simulink model "wlan_phy_rx_pmd"  (Simulink 7.8)
settings: Solver=VariableStepDiscrete  SolverMode=SingleTasking  StartTime=0.0  StopTime=simtime  RelTol=1e-3
PostLoadFcn:
  | wlan_phy_rx_init
InitFcn:
  | wlan_phy_rx_init
StartFcn:
  | wlan_phy_rx_init
values from neighbouring files (symbols this model uses):
  barker_seq20 = 0.95 * circshift(barker_seq20, [0 4]) ./ max(abs(barker_seq20))    [wlan_phy_rx_init.m]
  bit_scrambler_lfsr_bytes = bi2de(reshape(repmat(scr, 1, 8), 8, 127)', 'left-msb')    [wlan_phy_rx_init.m]
  deinterleave_ROM = [deinterleave_ROM interleave_64QAM zeros(1, 512-length(interleave_64QAM))]    [wlan_phy_rx_init.m]
  longCorr_coef_i = [3*real(LTS_corr)]    [wlan_phy_rx_init.m]
  longCorr_coef_q = [3*imag(LTS_corr)]    [wlan_phy_rx_init.m]
  MAX_CP_LEN = 32    [wlan_phy_rx_init.m]
  MAX_NCBPS = 288    [wlan_phy_rx_init.m]
  MAX_NUM_BYTES = 4096    [wlan_phy_rx_init.m]
  MAX_NUM_SC = 64    [wlan_phy_rx_init.m]
  MAX_NUM_SYMS = 600    [wlan_phy_rx_init.m]
  n_bits_preFFT_sampBuff = ceil(log2(4*MAX_NUM_SC))    [wlan_phy_rx_init.m]
  NCBPS_16QAM = 192    [wlan_phy_rx_init.m]
  NCBPS_64QAM = 288    [wlan_phy_rx_init.m]
  NCBPS_BPSK = 48    [wlan_phy_rx_init.m]
  NCBPS_QPSK = 96    [wlan_phy_rx_init.m]
  NDBPS_16QAM12 = 96    [wlan_phy_rx_init.m]
  NDBPS_16QAM34 = 144    [wlan_phy_rx_init.m]
  NDBPS_64QAM23 = 192    [wlan_phy_rx_init.m]
  NDBPS_64QAM34 = 216    [wlan_phy_rx_init.m]
  NDBPS_BPSK12 = 24    [wlan_phy_rx_init.m]
  NDBPS_BPSK34 = 36    [wlan_phy_rx_init.m]
  NDBPS_QPSK12 = 48    [wlan_phy_rx_init.m]
  NDBPS_QPSK34 = 72    [wlan_phy_rx_init.m]
  paylod_vec_samp_time = 8    [wlan_phy_rx_init.m]
  PHY_MIN_PKT_LEN = 14    [wlan_phy_rx_init.m]
  scr_ind_rev = zeros(1,128)    [wlan_phy_rx_init.m]
undefined symbols (not defined in the file or in its folder): gcbh, xlBlockLoadCallback, xlGetNormalizedPeriod, xlipgetpartsetting
libraries: xbsIndex_r4 x3519, simulink x1, xbsEDKLib_r4 x1
(part 8 of 21)

== system wlan_phy_rx_pmd/Chan Est & EQ/Chan Est/Q Estimate Buffer ==
  note: data
  Est_Data : Inport
  Est_Addr : Inport  Port=2
  Est_WE : Inport  Port=3
  First Sym : Inport  Port=4
  AddSub2 : xbsIndex_r4/AddSub  mode=Addition  use_carryin=off  use_carryout=off  en=off  latency=0  precision=User Defined  arith_type="Signed  (2's comp)"  n_bits=18  bin_pt=15  quantization=Truncate  overflow=Flag as error  dbl_ovrd=off  use_behavioral_HDL=on  hw_selection=Fabric  pipelined=off  use_rpm=on  block_type=addsub
      settings: xl_area=[0, 0, 0, 0, 0, 0, 0]  block_version=VER_STRING_GOES_HERE
  Constant Read State : xbsIndex_r4/Constant  const=0  gui_display_data_type=Fixed-point  arith_type=Boolean  n_bits=1  bin_pt=0  preci_type=Single  exp_width=8  frac_width=24  explicit_period=off  period=1  equ=P=C  opselect=C  inp2=PCIN>>17  opr=+  inp1=P  carry=CIN  dbl_ovrd=off  block_type=constant
      settings: block_version=VER_STRING_GOES_HERE
  Constant4 : xbsIndex_r4/Constant  const=0  gui_display_data_type=Fixed-point  arith_type=Signed (2's comp)  n_bits=18  bin_pt=15  preci_type=Single  exp_width=8  frac_width=24  explicit_period=off  period=1  equ=P=C  opselect=C  inp2=PCIN>>17  opr=+  inp1=P  carry=CIN  dbl_ovrd=off  block_type=constant
      settings: block_version=VER_STRING_GOES_HERE
  Convert1 : xbsIndex_r4/Convert  gui_display_data_type=Fixed-point  arith_type="Signed  (2's comp)"  n_bits=18  bin_pt=15  float_type=Single  exp_bits=8  fraction_bits=24  quantization=Truncate  overflow=Flag as error  en=off  latency=0  dbl_ovrd=off  pipeline=off  block_type=convert
      settings: xl_area=[0, 0, 0, 0, 0, 0, 0]  block_version=VER_STRING_GOES_HERE
  Convert3 : xbsIndex_r4/Convert  gui_display_data_type=Fixed-point  arith_type="Signed  (2's comp)"  n_bits=18  bin_pt=16  float_type=Single  exp_bits=8  fraction_bits=24  quantization=Truncate  overflow=Flag as error  en=off  latency=0  dbl_ovrd=off  pipeline=off  block_type=convert
      settings: xl_area=[0, 0, 0, 0, 0, 0, 0]  block_version=VER_STRING_GOES_HERE
  Delay3 : xbsIndex_r4/Delay  rst=off  infoeditControl=Selection of Reset will increase slice count due to use of real FFs and instead of SRLs  en=off  latency=1  dbl_ovrd=off  reg_retiming=off  block_type=delay
      settings: infoedit=Hardware notes: A delay line is a chain, each link of which is an SRL16 followed by a flip-flop. If register retiming is enabled, the delay line is a chain of flip-flops.  xl_area=[0, 0, 0, 0, 0, 0, 0]  block_version=VER_STRING_GOES_HERE
  Delay6 : xbsIndex_r4/Delay  rst=off  infoeditControl=Selection of Reset will increase slice count due to use of real FFs and instead of SRLs  en=off  latency=1  dbl_ovrd=off  reg_retiming=off  block_type=delay
      settings: infoedit=Hardware notes: A delay line is a chain, each link of which is an SRL16 followed by a flip-flop. If register retiming is enabled, the delay line is a chain of flip-flops.  xl_area=[0, 0, 0, 0, 0, 0, 0]  block_version=VER_STRING_GOES_HERE
  Delay7 : xbsIndex_r4/Delay  rst=off  infoeditControl=Selection of Reset will increase slice count due to use of real FFs and instead of SRLs  en=off  latency=1  dbl_ovrd=off  reg_retiming=off  block_type=delay
      settings: infoedit=Hardware notes: A delay line is a chain, each link of which is an SRL16 followed by a flip-flop. If register retiming is enabled, the delay line is a chain of flip-flops.  xl_area=[0, 0, 0, 0, 0, 0, 0]  block_version=VER_STRING_GOES_HERE
  Dual Port RAM : xbsIndex_r4/Dual Port RAM  depth=MAX_NUM_SC  initVector=0  distributed_mem=Block RAM  init_a=0  init_b=0  rst_a=off  rst_b=on  en_a=off  en_b=off  latency=1  write_mode_A=Read After Write  write_mode_B=Read After Write  dbl_ovrd=off  optimize=Area  block_type=dpram
      settings: xl_area=[0, 0, 0, 0, 0, 0, 0]  block_version=VER_STRING_GOES_HERE
  Gateway Out{1..8} (8 alike) : xbsIndex_r4/Gateway Out  inherit_from_input=off  hdl_port=off  timing_constraint=None  locs_specified=off  LOCs={}  UseAsDAC=off  DACChannel='1'  block_type=gatewayout
  H est buff : Scope  NumInputPorts=8
      settings: TimeRange=14000  YMin=0~-0.1~-0.2~-0.4~0~0~0~-0.2  YMax=80~0.1~0.2~0.4~80~1~1~0.2  SaveName=ScopeData7
  h[n] : Outport
  wires:
  Gateway Out7/1 -> H est buff/8  "Port A Out"
  Dual Port RAM/1 -> Gateway Out7/1, Convert3/1
  Convert3/1 -> h[n]/1
  Gateway Out6/1 -> H est buff/7  "Reset"
  Gateway Out5/1 -> H est buff/6  "Est We"
  Gateway Out4/1 -> H est buff/5  "Est Addr"
  Gateway Out3/1 -> H est buff/4  "Port A In"
  Gateway Out2/1 -> H est buff/3  "Port B Out"
  Gateway Out1/1 -> H est buff/2  "H Est"
  Gateway Out8/1 -> H est buff/1  "Addr A"
  Dual Port RAM/2 -> Gateway Out2/1, Convert1/1
  Est_Data/1 -> Delay7/1
  First Sym/1 -> Gateway Out6/1, Dual Port RAM/7
  Est_Addr/1 -> Gateway Out4/1, Dual Port RAM/4, Delay6/1
  Est_WE/1 -> Delay3/1
  Delay7/1 -> Gateway Out1/1, AddSub2/2
  Delay3/1 -> Gateway Out5/1, Dual Port RAM/3
  Delay6/1 -> Gateway Out8/1, Dual Port RAM/1
  Convert1/1 -> AddSub2/1
  AddSub2/1 -> Gateway Out3/1, Dual Port RAM/2
  Constant Read State/1 -> Dual Port RAM/6
  Constant4/1 -> Dual Port RAM/5

== system wlan_phy_rx_pmd/Chan Est & EQ/Chan Est/To Float ==
  "x_I " : Inport
  "x_Q " : Inport  Port=2
  "H_I " : Inport  Port=3
  "H_Q " : Inport  Port=4
  x_ind : Inport  Port=5
  x_valid : Inport  Port=6
  sym_ind : Inport  Port=7
  Delay1 : xbsIndex_r4/Delay  rst=off  infoeditControl=Selection of Reset will increase slice count due to use of real FFs and instead of SRLs  en=off  latency=3  dbl_ovrd=off  reg_retiming=off  block_type=delay
  Delay2 : xbsIndex_r4/Delay  rst=off  infoeditControl=Selection of Reset will increase slice count due to use of real FFs and instead of SRLs  en=off  latency=3  dbl_ovrd=off  reg_retiming=off  block_type=delay
  Delay3 : xbsIndex_r4/Delay  rst=off  infoeditControl=Selection of Reset will increase slice count due to use of real FFs and instead of SRLs  en=off  latency=3  dbl_ovrd=off  reg_retiming=off  block_type=delay
  Inverter : xbsIndex_r4/Inverter  en=off  latency=0  dbl_ovrd=off  block_type=inv
  Register{,1..3} (4 alike) : xbsIndex_r4/Register  init=0  rst=on  en=off  dbl_ovrd=off  block_type=register
  To FP{1..4} (4 alike) : xbsIndex_r4/Convert  gui_display_data_type=Floating-point  arith_type=Floating-point  n_bits=16  bin_pt=14  float_type=Single  exp_bits=8  fraction_bits=24  quantization=Truncate  overflow=Wrap  en=off  latency=2  dbl_ovrd=off  pipeline=off  block_type=convert
  " x_I" : Outport
  " x_Q" : Outport  Port=2
  H_I : Outport  Port=3
  " H_Q" : Outport  Port=4
  " x_ind" : Outport  Port=5
  " x_valid" : Outport  Port=6
  " sym_ind" : Outport  Port=7
  wires:
  To FP3/1 -> " H_Q"/1
  To FP2/1 -> H_I/1
  To FP1/1 -> " x_Q"/1
  To FP4/1 -> " x_I"/1
  Register3/1 -> To FP3/1
  Register2/1 -> To FP2/1
  Inverter/1 -> Register3/2, Register2/2, Register1/2, Register/2
  Register1/1 -> To FP1/1
  Register/1 -> To FP4/1
  "x_I "/1 -> Register/1
  "x_Q "/1 -> Register1/1
  "H_I "/1 -> Register2/1
  "H_Q "/1 -> Register3/1
  x_valid/1 -> Inverter/1, Delay1/1
  Delay1/1 -> " x_valid"/1
  x_ind/1 -> Delay2/1
  Delay2/1 -> " x_ind"/1
  sym_ind/1 -> Delay3/1
  Delay3/1 -> " sym_ind"/1

== system wlan_phy_rx_pmd/Chan Est & EQ/EQ & Pilot Processing/Debug Outputs ==
  note: Only capture data and pilot subcarriers
      | for debug outputs and chipscope
  I : Inport
  Q : Inport  Port=2
  valid : Inport  Port=3
  x_ind : Inport  Port=4
  4x Buffer : Subsystem
  Constant : xbsIndex_r4/Constant  const=0  gui_display_data_type=Fixed-point  arith_type=Unsigned  n_bits=1  bin_pt=0  preci_type=Single  exp_width=8  frac_width=24  explicit_period=off  period=1  equ=P=C  opselect=C  inp2=PCIN>>17  opr=+  inp1=P  carry=CIN  dbl_ovrd=off  block_type=constant
  Constant1 : xbsIndex_r4/Constant  const=27  gui_display_data_type=Fixed-point  arith_type=Unsigned  n_bits=5  bin_pt=0  preci_type=Single  exp_width=8  frac_width=24  explicit_period=off  period=1  equ=P=C  opselect=C  inp2=PCIN>>17  opr=+  inp1=P  carry=CIN  dbl_ovrd=off  block_type=constant
  Constant2 : xbsIndex_r4/Constant  const=37  gui_display_data_type=Fixed-point  arith_type=Unsigned  n_bits=6  bin_pt=0  preci_type=Single  exp_width=8  frac_width=24  explicit_period=off  period=1  equ=P=C  opselect=C  inp2=PCIN>>17  opr=+  inp1=P  carry=CIN  dbl_ovrd=off  block_type=constant
  Debug EQ IQ : Scope  NumInputPorts=7
      settings: YMin=-1.1~-1~-1.1~-1~-5~-5~-5  YMax=1.1~1~1.2~1~5~5~5  SaveName=ScopeData13
  Delay : xbsIndex_r4/Delay  rst=off  infoeditControl=Selection of Reset will increase slice count due to use of real FFs and instead of SRLs  en=off  latency=1  dbl_ovrd=off  reg_retiming=off  block_type=delay
  Delay1 : xbsIndex_r4/Delay  rst=off  infoeditControl=Selection of Reset will increase slice count due to use of real FFs and instead of SRLs  en=off  latency=1  dbl_ovrd=off  reg_retiming=off  block_type=delay
  Gateway Out12 : xbsIndex_r4/Gateway Out  inherit_from_input=off  hdl_port=off  timing_constraint=None  locs_specified=off  LOCs={}  UseAsDAC=off  DACChannel='1'  block_type=gatewayout
  Gateway Out13 : xbsIndex_r4/Gateway Out  inherit_from_input=off  hdl_port=off  timing_constraint=None  locs_specified=off  LOCs={}  UseAsDAC=off  DACChannel='1'  block_type=gatewayout
  Gateway Out8 : xbsIndex_r4/Gateway Out  inherit_from_input=off  hdl_port=off  timing_constraint=None  locs_specified=off  LOCs={}  UseAsDAC=off  DACChannel='1'  block_type=gatewayout
  Inverter1 : xbsIndex_r4/Inverter  en=off  latency=0  dbl_ovrd=off  block_type=inv
  Logical : xbsIndex_r4/Logical  logical_function=AND  inputs=3  en=off  latency=0  precision=Full  arith_type=Unsigned  n_bits=16  bin_pt=0  align_bp=on  dbl_ovrd=off  block_type=logical
  Logical1 : xbsIndex_r4/Logical  logical_function=OR  inputs=2  en=off  latency=0  precision=Full  arith_type=Unsigned  n_bits=16  bin_pt=0  align_bp=on  dbl_ovrd=off  block_type=logical
  Real-Imag to Complex : RealImagToComplex
  Register{,1..2,7..8} (5 alike) : xbsIndex_r4/Register  init=0  rst=off  en=off  dbl_ovrd=off  block_type=register
  Register{3..6} (4 alike) : xbsIndex_r4/Register  init=0  rst=on  en=off  dbl_ovrd=off  block_type=register
  Relational : xbsIndex_r4/Relational  mode=a!=b  en=off  latency=0  dbl_ovrd=off  block_type=relational
  Relational1 : xbsIndex_r4/Relational  mode=a<b  en=off  latency=0  dbl_ovrd=off  block_type=relational
  Relational2 : xbsIndex_r4/Relational  mode=a>b  en=off  latency=0  dbl_ovrd=off  block_type=relational
  To FP1 : xbsIndex_r4/Convert  gui_display_data_type=Fixed-point  arith_type="Signed  (2's comp)"  n_bits=14  bin_pt=13  float_type=Single  exp_bits=8  fraction_bits=24  quantization=Truncate  overflow=Saturate  en=off  latency=0  dbl_ovrd=off  pipeline=off  block_type=convert
  To FP4 : xbsIndex_r4/Convert  gui_display_data_type=Fixed-point  arith_type="Signed  (2's comp)"  n_bits=14  bin_pt=13  float_type=Single  exp_bits=8  fraction_bits=24  quantization=Truncate  overflow=Saturate  en=off  latency=0  dbl_ovrd=off  pipeline=off  block_type=convert
  To Workspace : ToWorkspace  VariableName=eq_out  SampleTime=-1  SaveFormat=Array
  To Workspace1 : ToWorkspace  VariableName=eq_out_valid  SampleTime=-1  SaveFormat=Array
  XY Graph : simulink/Sinks/XY Graph  xmin=-1.2  xmax=1.2  ymin=-1.2  ymax=1.2  st=1
  dbg_EQ_I : xbsIndex_r4/Gateway Out  inherit_from_input=off  hdl_port=on  timing_constraint=None  locs_specified=off  LOCs={}  UseAsDAC=off  DACChannel='1'  block_type=gatewayout
  dbg_EQ_Q : xbsIndex_r4/Gateway Out  inherit_from_input=off  hdl_port=on  timing_constraint=None  locs_specified=off  LOCs={}  UseAsDAC=off  DACChannel='1'  block_type=gatewayout
  dbg_EQ_Q{1..4} (4 alike) : xbsIndex_r4/Gateway Out  inherit_from_input=off  hdl_port=off  timing_constraint=None  locs_specified=off  LOCs={}  UseAsDAC=off  DACChannel='1'  block_type=gatewayout
  tag scope: CS_EQ_I=global, CS_EQ_Q=global, CS_EQ_Valid=global
  wires:
  Inverter1/1 -> Register3/2, Register6/2
  Logical/1 -> Inverter1/1, Delay/1, Gateway Out8/1, 4x Buffer/3(Vin)
  Register2/1 -> To FP1/1, Register6/1, Gateway Out13/1
  Register1/1 -> To FP4/1, Register3/1, Gateway Out12/1
  x_ind/1 -> Register/1
  Register/1 -> Relational/1, Relational1/1, Relational2/1
  Constant/1 -> Relational/2
  Relational/1 -> Logical/2
  I/1 -> Register1/1
  Q/1 -> Register2/1
  Constant1/1 -> Relational1/2
  Constant2/1 -> Relational2/2
  Relational1/1 -> Logical1/1
  Relational2/1 -> Logical1/2
  Logical1/1 -> Logical/3
  Register3/1 -> [CS_EQ_I], dbg_EQ_Q3/1
  Register6/1 -> [CS_EQ_Q], dbg_EQ_Q1/1
  Delay/1 -> [CS_EQ_Valid], dbg_EQ_Q2/1
  valid/1 -> Delay1/1
  Delay1/1 -> Logical/1
  Register7/1 -> dbg_EQ_I/1
  Register8/1 -> dbg_EQ_Q/1
  dbg_EQ_I/1 -> XY Graph/1, Debug EQ IQ/3
  dbg_EQ_Q/1 -> XY Graph/2, Debug EQ IQ/4
  dbg_EQ_Q3/1 -> Debug EQ IQ/5
  dbg_EQ_Q1/1 -> Debug EQ IQ/6
  dbg_EQ_Q2/1 -> Debug EQ IQ/7
  Gateway Out12/1 -> Real-Imag to Complex/1
  Gateway Out13/1 -> Real-Imag to Complex/2
  Real-Imag to Complex/1 -> To Workspace/1
  Gateway Out8/1 -> To Workspace1/1
  To FP4/1 -> 4x Buffer/1(I)
  To FP1/1 -> 4x Buffer/2(Q)
  Register4/1 -> Register7/1
  4x Buffer/1(" I") -> Register4/1
  4x Buffer/3(#Vout) -> Register4/2, Register5/2, dbg_EQ_Q4/1
  4x Buffer/2(" Q") -> Register5/1
  Register5/1 -> Register8/1
  dbg_EQ_Q4/1 -> Debug EQ IQ/2

== system wlan_phy_rx_pmd/Chan Est & EQ/EQ & Pilot Processing/Mag ==
  I : Inport
  Q : Inport  Port=2
  AddSub : xbsIndex_r4/AddSub  mode=Addition  use_carryin=off  use_carryout=off  en=off  latency=4  precision=Full  arith_type=Unsigned  n_bits=16  bin_pt=14  quantization=Truncate  overflow=Wrap  dbl_ovrd=off  use_behavioral_HDL=off  hw_selection=DSP48  pipelined=off  block_type=addsub
  Mult : xbsIndex_r4/Mult  precision=Full  arith_type="Signed  (2's comp)"  n_bits=16  bin_pt=14  quantization=Truncate  overflow=Wrap  en=off  latency=2  dbl_ovrd=off  use_behavioral_HDL=off  opt=Speed  use_embedded=on  optimum_pipeline=off  pipeline=on  use_rpm=on  placement_style=Triangular  block_type=mult
  Mult1 : xbsIndex_r4/Mult  precision=Full  arith_type="Signed  (2's comp)"  n_bits=16  bin_pt=14  quantization=Truncate  overflow=Wrap  en=off  latency=2  dbl_ovrd=off  use_behavioral_HDL=off  opt=Speed  use_embedded=on  optimum_pipeline=off  pipeline=on  use_rpm=on  placement_style=Triangular  block_type=mult
  Register1 : xbsIndex_r4/Register  init=0  rst=off  en=off  dbl_ovrd=off  block_type=register
  Register2 : xbsIndex_r4/Register  init=0  rst=off  en=off  dbl_ovrd=off  block_type=register
  Register3 : xbsIndex_r4/Register  init=0  rst=off  en=off  dbl_ovrd=off  block_type=register
  M : Outport
  wires:
  I/1 -> Mult/1, Mult/2
  Q/1 -> Mult1/1, Mult1/2
  Mult/1 -> Register2/1
  Mult1/1 -> Register1/1
  AddSub/1 -> Register3/1
  Register2/1 -> AddSub/1
  Register1/1 -> AddSub/2
  Register3/1 -> M/1

== system wlan_phy_rx_pmd/Chan Est & EQ/EQ & Pilot Processing/Phase Correction ==
  x_I : Inport
  x_Q : Inport  Port=2
  Phase : Inport  Port=3
  x_valid : Inport  Port=4
  "CORDIC 5.0 " : xbsIndex_r4/CORDIC 5.0   functional_selection=Rotate  architectural_configuration=Parallel  pipelining_mode=Optimal  data_format=SignedFraction  phase_format=Scaled_Radians  input_width=16  output_width=18  round_mode=Truncate  iterations=0  precision=0  compensation_scaling=Embedded_Multiplier  coarse_rotation=on  aclken=off  aresetn=off  out_tready=off  cartesian_has_tuser=off  cartesian_has_tlast=off  cartesian_tuser_width=1  phase_has_tuser=off  phase_has_tlast=off  phase_tuser_width=1  out_tlast_behv=Null  flow_control=NonBlocking  optimize_goal=Performance  trim_axipin_name=on  ip_name=CORDIC  ip_version=5.0  dsptool_ready=true  ipcore_usecache=true  ipcore_useipmodelcache=true  wrapper_available=true  port_translation_map={ 'aclken' => 'en', 'aresetn' => 'rst' }  block_type=cordic_v5_0
      settings: infoedit=CORDIC 5.0
  Constant1 : xbsIndex_r4/Constant  const=1  gui_display_data_type=Boolean  arith_type=Boolean  n_bits=16  bin_pt=13  preci_type=Single  exp_width=8  frac_width=24  explicit_period=on  period=1  equ=P=C  opselect=C  inp2=PCIN>>17  opr=+  inp1=P  carry=CIN  dbl_ovrd=off  block_type=constant
  Convert : xbsIndex_r4/Convert  gui_display_data_type=Fixed-point  arith_type="Signed  (2's comp)"  n_bits=16  bin_pt=13  float_type=Single  exp_bits=8  fraction_bits=24  quantization=Truncate  overflow=Flag as error  en=off  latency=0  dbl_ovrd=off  pipeline=off  block_type=convert
  Gateway Out{,1..4,6..7} (7 alike) : xbsIndex_r4/Gateway Out  inherit_from_input=off  hdl_port=off  timing_constraint=None  locs_specified=off  LOCs={}  UseAsDAC=off  DACChannel='1'  block_type=gatewayout
  Negate : xbsIndex_r4/Negate  precision=Full  arith_type=Unsigned  n_bits=16  bin_pt=14  quantization=Truncate  overflow=Wrap  en=off  latency=0  dbl_ovrd=off  block_type=negate
  Phase Correct : Scope  NumInputPorts=8
      settings: TimeRange=10000  YMin=0~-2~-2~-1~0.27~0~-1.5~-1  YMax=1~1~1.5~1~0.34~1~1.5~0.75  SaveName=ScopeData11
  " x_I" : Outport
  " x_Q" : Outport  Port=2
  " x_valid" : Outport  Port=3
  tag scope: CS_Phase_Err=global
  wires:
  Phase/1 -> Negate/1, [CS_Phase_Err], Gateway Out6/1
  Gateway Out6/1 -> Phase Correct/5  "Phase"
  Gateway Out4/1 -> Phase Correct/3  "X_Q"
  Gateway Out2/1 -> Phase Correct/7  "I Out"
  Gateway Out1/1 -> Phase Correct/2  "X_I"
  Gateway Out/1 -> Phase Correct/1  "X_IQ Valid"
  Gateway Out7/1 -> Phase Correct/6  "Valid Out"
  Gateway Out3/1 -> Phase Correct/8  "Q Out"
  x_I/1 -> Gateway Out1/1, "CORDIC 5.0 "/3
  x_Q/1 -> Gateway Out4/1, "CORDIC 5.0 "/2
  x_valid/1 -> Gateway Out/1, "CORDIC 5.0 "/1
  Convert/1 -> "CORDIC 5.0 "/5
  "CORDIC 5.0 "/1 -> Gateway Out7/1, " x_valid"/1
  "CORDIC 5.0 "/2 -> Gateway Out3/1, " x_Q"/1
  "CORDIC 5.0 "/3 -> Gateway Out2/1, " x_I"/1
  Constant1/1 -> "CORDIC 5.0 "/4
  Negate/1 -> Convert/1

== system wlan_phy_rx_pmd/Chan Est & EQ/EQ & Pilot Processing/Phase Err Calc ==
  CP_I : Inport
  CP_Q : Inport  Port=2
  x_valid : Inport  Port=3
  x_ind : Inport  Port=4
  sym_ind : Inport  Port=5
  Accum1 : Subsystem
  Accum2 : Subsystem
  Constant3 : xbsIndex_r4/Constant  const=0  gui_display_data_type=Fixed-point  arith_type=Unsigned  n_bits=6  bin_pt=0  preci_type=Single  exp_width=8  frac_width=24  explicit_period=off  period=8  equ=P=C  opselect=C  inp2=PCIN>>17  opr=+  inp1=P  carry=CIN  dbl_ovrd=off  block_type=constant
  Count : Subsystem
  Delay8 : xbsIndex_r4/Delay  rst=off  infoeditControl=Selection of Reset will increase slice count due to use of real FFs and instead of SRLs  en=off  latency=1  dbl_ovrd=off  reg_retiming=off  block_type=delay
  Descramble : Subsystem
  Gateway Out{3..10} (8 alike) : xbsIndex_r4/Gateway Out  inherit_from_input=off  hdl_port=off  timing_constraint=None  locs_specified=off  LOCs={}  UseAsDAC=off  DACChannel='1'  block_type=gatewayout
  Phase Calc : Subsystem
  Pilot Proc : Scope  NumInputPorts=10
      settings: TimeRange=14000  YMin=0~-0.1~-0.2~-0.4~0~0~0~-0.2~-5~-5  YMax=80~0.1~0.2~0.4~80~1~1~0.2~5~5  SaveName=ScopeData10
  Pilot Selection : Subsystem
  Register1 : xbsIndex_r4/Register  init=0  rst=off  en=on  dbl_ovrd=off  block_type=register
  Register2 : xbsIndex_r4/Register  init=0  rst=off  en=on  dbl_ovrd=off  block_type=register
  Relational1 : xbsIndex_r4/Relational  mode=a=b  en=off  latency=0  dbl_ovrd=off  block_type=relational
      settings: xl_area=[2 0 0 3 0 0 0]  block_version=VER_STRING_GOES_HERE
  Relational7 : xbsIndex_r4/Relational  mode=a=b  en=off  latency=0  dbl_ovrd=off  block_type=relational
      settings: xl_area=[2 0 0 3 0 0 0]  block_version=VER_STRING_GOES_HERE
  Sym Phase Err : Outport
  wires:
  Relational7/1 -> Count/2(Rst), Accum1/3(Rst), Accum2/3(Rst)
  Accum1/1(Sum) -> Gateway Out3/1, Phase Calc/1(I)
  Accum2/1(Sum) -> Gateway Out4/1, Phase Calc/2(Q)
  Descramble/1(" x_I") -> Accum1/2(D), Register1/1
  Descramble/2(" x_Q") -> Register2/1, Accum2/2(D)
  Delay8/1 -> Accum1/1(En), Count/1(En), Accum2/1(En), Register2/2, Register1/2
  Count/1(Last) -> Phase Calc/3(IQ Valid)
  Pilot Selection/1(Pilot) -> Delay8/1
  Pilot Selection/2(Negate) -> Descramble/1(Negate)
  CP_I/1 -> Descramble/2(x_I), Gateway Out9/1
  CP_Q/1 -> Descramble/3(x_Q), Gateway Out10/1
  Phase Calc/1(phase err) -> Sym Phase Err/1, Gateway Out5/1
  sym_ind/1 -> Descramble/4(Sym Ind), Relational1/1
  Relational1/1 -> Phase Calc/4(Reset)
  x_valid/1 -> Pilot Selection/1(x_valid)
  x_ind/1 -> Pilot Selection/2(x_ind), Relational7/1, Gateway Out8/1
  Constant3/1 -> Relational7/2, Relational1/2
  Gateway Out8/1 -> Pilot Proc/1  "X Ind"
  Gateway Out3/1 -> Pilot Proc/4  "Accum I"
  Gateway Out4/1 -> Pilot Proc/5  "Accum Q"
  Gateway Out5/1 -> Pilot Proc/6  "Phase Err"
  Gateway Out6/1 -> Pilot Proc/7  "Descram I"
  Gateway Out7/1 -> Pilot Proc/8  "Descram Q"
  Gateway Out9/1 -> Pilot Proc/9  "Conj Prod I"
  Gateway Out10/1 -> Pilot Proc/10  "Conj Prod Q"
  Register1/1 -> Gateway Out6/1
  Register2/1 -> Gateway Out7/1

== system wlan_phy_rx_pmd/Chan Est & EQ/EQ & Pilot Processing/a*conj(b) ==
  note: Implements A*conj(B)
  A_I : Inport
  A_Q : Inport  Port=2
  B_I : Inport  Port=3
  B_Q : Inport  Port=4
  AddSub : xbsIndex_r4/AddSub  mode=Addition  use_carryin=off  use_carryout=off  en=off  latency=4  precision=Full  arith_type=Unsigned  n_bits=16  bin_pt=14  quantization=Truncate  overflow=Wrap  dbl_ovrd=off  use_behavioral_HDL=off  hw_selection=DSP48  pipelined=off  block_type=addsub
  AddSub1 : xbsIndex_r4/AddSub  mode=Subtraction  use_carryin=off  use_carryout=off  en=off  latency=4  precision=Full  arith_type=Unsigned  n_bits=16  bin_pt=14  quantization=Truncate  overflow=Wrap  dbl_ovrd=off  use_behavioral_HDL=off  hw_selection=DSP48  pipelined=off  block_type=addsub
  Mult{1..4} (4 alike) : xbsIndex_r4/Mult  precision=Full  arith_type="Signed  (2's comp)"  n_bits=16  bin_pt=14  quantization=Truncate  overflow=Wrap  en=off  latency=2  dbl_ovrd=off  use_behavioral_HDL=off  opt=Speed  use_embedded=on  optimum_pipeline=off  pipeline=on  use_rpm=on  placement_style=Triangular  block_type=mult
  Register{1..6} (6 alike) : xbsIndex_r4/Register  init=0  rst=off  en=off  dbl_ovrd=off  block_type=register
  P_I : Outport
  P_Q : Outport  Port=2
  wires:
  A_I/1 -> Mult1/1, Mult4/2
  B_I/1 -> Mult1/2, Mult3/2
  A_Q/1 -> Mult2/1, Mult3/1
  B_Q/1 -> Mult2/2, Mult4/1
  Mult1/1 -> Register2/1
  Mult2/1 -> Register1/1
  Mult3/1 -> Register3/1
  Mult4/1 -> Register4/1
  AddSub/1 -> Register5/1
  AddSub1/1 -> Register6/1
  Register2/1 -> AddSub/1
  Register1/1 -> AddSub/2
  Register3/1 -> AddSub1/1
  Register4/1 -> AddSub1/2
  Register5/1 -> P_I/1
  Register6/1 -> P_Q/1

== system wlan_phy_rx_pmd/DSSS Rx/Demod/Bit-to-Byte ==
  Bit : Inport
  Bit Valid : Inport  Port=2
  Concat : xbsIndex_r4/Concat  num_inputs=8  dbl_ovrd=off  block_type=concat
  Constant : xbsIndex_r4/Constant  const=8  gui_display_data_type=Fixed-point  arith_type=Unsigned  n_bits=4  bin_pt=0  preci_type=Single  exp_width=8  frac_width=24  explicit_period=off  period=1  equ=P=C  opselect=C  inp2=PCIN>>17  opr=+  inp1=P  carry=CIN  dbl_ovrd=off  block_type=constant
  Counter : xbsIndex_r4/Counter  cnt_type=Free Running  cnt_to=Inf  operation=Up  start_count=0  cnt_by_val=1  arith_type=Unsigned  n_bits=8  bin_pt=0  load_pin=off  rst=on  en=on  explicit_period=off  period=1  dbl_ovrd=off  use_behavioral_HDL=off  implementation=Fabric  block_type=counter
  Counter1 : xbsIndex_r4/Counter  cnt_type=Free Running  cnt_to=Inf  operation=Up  start_count=0  cnt_by_val=1  arith_type=Unsigned  n_bits=ceil(log2(MAX_NUM_BYTES))  bin_pt=0  load_pin=off  rst=on  en=on  explicit_period=off  period=1  dbl_ovrd=off  use_behavioral_HDL=off  implementation=Fabric  block_type=counter
  Delay : xbsIndex_r4/Delay  rst=off  infoeditControl=Selection of Reset will increase slice count due to use of real FFs and instead of SRLs  en=off  latency=1  dbl_ovrd=off  reg_retiming=off  block_type=delay
  Delay1 : xbsIndex_r4/Delay  rst=off  infoeditControl=Selection of Reset will increase slice count due to use of real FFs and instead of SRLs  en=off  latency=1  dbl_ovrd=off  reg_retiming=off  block_type=delay
  Logical1 : xbsIndex_r4/Logical  logical_function=OR  inputs=2  en=off  latency=0  precision=Full  arith_type=Unsigned  n_bits=8  bin_pt=2  align_bp=on  dbl_ovrd=off  block_type=logical
      settings: xl_area=[1 0 0 1 0 0 0]  block_version=VER_STRING_GOES_HERE
  Register{,1..8} (9 alike) : xbsIndex_r4/Register  init=0  rst=on  en=on  dbl_ovrd=off  block_type=register
  Relational : xbsIndex_r4/Relational  mode=a=b  en=off  latency=0  dbl_ovrd=off  block_type=relational
  Byte : Outport
  Byte Valid : Outport  Port=2
  Byte Ind : Outport  Port=3
  wires:
  Register/1 -> Register1/1, Concat/1
  Register1/1 -> Register2/1, Concat/2
  Register2/1 -> Register3/1, Concat/3
  Register3/1 -> Register4/1, Concat/4
  Register4/1 -> Register5/1, Concat/5
  Register5/1 -> Register6/1, Concat/6
  Register6/1 -> Register7/1, Concat/7
  Bit Valid/1 -> Register1/3, Register2/3, Register3/3, Register4/3, Register5/3, Register6/3, Register7/3, Register/3, Counter/2
  [DSSS_RX_RESET] -> Register6/2, Register7/2, Register5/2, Register4/2, Register3/2, Register2/2, Register1/2, Register/2, Logical1/2
  Bit/1 -> Register/1
  Register7/1 -> Concat/8
  Concat/1 -> Register8/1
  Constant/1 -> Relational/2
  Counter/1 -> Relational/1
  Logical1/1 -> Counter/1
  Relational/1 -> Logical1/1, Register8/3, Delay1/1, Delay/1
  [DSSS_RX_RESET] -> Register8/2
  Register8/1 -> Byte/1
  Delay/1 -> Byte Valid/1
  [DSSS_RX_RESET] -> Counter1/1
  Counter1/1 -> Byte Ind/1
  Delay1/1 -> Counter1/2

== system wlan_phy_rx_pmd/DSSS Rx/Demod/DBPSK Demod ==
  IQ Valid : Inport
  I : Inport  Port=2
  Q : Inport  Port=3
  Absolute : xbsIndex_r4/Absolute  precision=Full  arith_type=Unsigned  n_bits=16  bin_pt=14  quantization=Truncate  overflow=Wrap  en=off  latency=0  dbl_ovrd=off  block_type=abs
  AddSub : xbsIndex_r4/AddSub  mode=Subtraction  use_carryin=off  use_carryout=off  en=off  latency=0  precision=Full  arith_type=Unsigned  n_bits=16  bin_pt=14  quantization=Truncate  overflow=Wrap  dbl_ovrd=off  use_behavioral_HDL=off  hw_selection=Fabric  pipelined=off  block_type=addsub
  "CORDIC 5.0 " : xbsIndex_r4/CORDIC 5.0   functional_selection=Arc_Tan  architectural_configuration=Parallel  pipelining_mode=Maximum  data_format=SignedFraction  phase_format=Scaled_Radians  input_width=16  output_width=16  round_mode=Truncate  iterations=0  precision=0  compensation_scaling=No_Scale_Compensation  coarse_rotation=on  aclken=off  aresetn=off  out_tready=off  cartesian_has_tuser=off  cartesian_has_tlast=off  cartesian_tuser_width=1  phase_has_tuser=off  phase_has_tlast=off  phase_tuser_width=1  out_tlast_behv=Null  flow_control=NonBlocking  optimize_goal=Resources  trim_axipin_name=on  ip_name=CORDIC  ip_version=5.0  dsptool_ready=true  ipcore_usecache=true  ipcore_useipmodelcache=true  wrapper_available=true  port_translation_map={ 'aclken' => 'en', 'aresetn' => 'rst' }  block_type=cordic_v5_0
      settings: infoedit=CORDIC 5.0
  Constant : xbsIndex_r4/Constant  const=0.5  gui_display_data_type=Fixed-point  arith_type=Signed (2's comp)  n_bits=4  bin_pt=3  preci_type=Single  exp_width=8  frac_width=24  explicit_period=off  period=1  equ=P=C  opselect=C  inp2=PCIN>>17  opr=+  inp1=P  carry=CIN  dbl_ovrd=off  block_type=constant
  Constant1 : xbsIndex_r4/Constant  const=1.5  gui_display_data_type=Fixed-point  arith_type=Signed (2's comp)  n_bits=6  bin_pt=3  preci_type=Single  exp_width=8  frac_width=24  explicit_period=off  period=1  equ=P=C  opselect=C  inp2=PCIN>>17  opr=+  inp1=P  carry=CIN  dbl_ovrd=off  block_type=constant
  Convert : xbsIndex_r4/Convert  gui_display_data_type=Fixed-point  arith_type=Unsigned  n_bits=1  bin_pt=0  float_type=Single  exp_bits=8  fraction_bits=24  quantization=Truncate  overflow=Wrap  en=off  latency=0  dbl_ovrd=off  pipeline=off  block_type=convert
  Gateway Out2 : xbsIndex_r4/Gateway Out  inherit_from_input=off  hdl_port=off  timing_constraint=None  locs_specified=off  LOCs={}  UseAsDAC=off  DACChannel='1'  block_type=gatewayout
  Gateway Out3 : xbsIndex_r4/Gateway Out  inherit_from_input=off  hdl_port=off  timing_constraint=None  locs_specified=off  LOCs={}  UseAsDAC=off  DACChannel='1'  block_type=gatewayout
  Gateway Out6 : xbsIndex_r4/Gateway Out  inherit_from_input=off  hdl_port=off  timing_constraint=None  locs_specified=off  LOCs={}  UseAsDAC=off  DACChannel='1'  block_type=gatewayout
  Logical : xbsIndex_r4/Logical  logical_function=AND  inputs=2  en=off  latency=0  precision=Full  arith_type=Unsigned  n_bits=16  bin_pt=0  align_bp=on  dbl_ovrd=off  block_type=logical
  Phase Cmp : Scope  NumInputPorts=7
      settings: TimeRange=80000  YMin=-1~-0.5~0~-0.1~5~0~0  YMax=1~0.3~1~1.1~40~1~300  SaveName=ScopeData6
  Register{,1..3} (4 alike) : xbsIndex_r4/Register  init=0  rst=off  en=off  dbl_ovrd=off  block_type=register
  Register4 : xbsIndex_r4/Register  init=0  rst=off  en=on  dbl_ovrd=off  block_type=register
  Relational : xbsIndex_r4/Relational  mode=a>b  en=off  latency=0  dbl_ovrd=off  block_type=relational
  Relational1 : xbsIndex_r4/Relational  mode=a<b  en=off  latency=0  dbl_ovrd=off  block_type=relational
  bit : Outport
  bit valid : Outport  Port=2
  wires:
  IQ Valid/1 -> "CORDIC 5.0 "/1
  I/1 -> "CORDIC 5.0 "/2
  Q/1 -> "CORDIC 5.0 "/3
  "CORDIC 5.0 "/2 -> Register1/1
  "CORDIC 5.0 "/1 -> Register/1
  Register4/1 -> AddSub/2, Gateway Out3/1
  AddSub/1 -> Absolute/1
  Constant/1 -> Relational/2
  Absolute/1 -> Gateway Out6/1, Relational/1, Relational1/1
  Relational/1 -> Logical/1
  Convert/1 -> Register2/1
  Gateway Out2/1 -> Phase Cmp/1
  Gateway Out3/1 -> Phase Cmp/2
  Gateway Out6/1 -> Phase Cmp/3
  Constant1/1 -> Relational1/2
  Relational1/1 -> Logical/2
  Logical/1 -> Convert/1
  Register/1 -> Register3/1, Register4/2
  Register1/1 -> AddSub/1, Gateway Out2/1, Register4/1
  Register2/1 -> bit/1
  Register3/1 -> bit valid/1

== system wlan_phy_rx_pmd/DSSS Rx/Demod/De-spread ==
  I : Inport
  Q : Inport  Port=2
  Sym Start : Inport  Port=3
  Accumulator : xbsIndex_r4/Accumulator  operation=Add  n_bits=22  overflow=Wrap  scale=1  rst=on  infoeditControl=reset for floating point data type must be asserted for a minimum of 2 cycles  hasbypass=on  en=off  latency=0  dbl_ovrd=off  msb_inp=100  msb=100  lsb=-100  use_behavioral_HDL=on  implementation=Fabric  block_type=accum
  Accumulator1 : xbsIndex_r4/Accumulator  operation=Add  n_bits=22  overflow=Wrap  scale=1  rst=on  infoeditControl=reset for floating point data type must be asserted for a minimum of 2 cycles  hasbypass=on  en=off  latency=0  dbl_ovrd=off  msb_inp=100  msb=100  lsb=-100  use_behavioral_HDL=on  implementation=Fabric  block_type=accum
  Constant : xbsIndex_r4/Constant  const=0  gui_display_data_type=Fixed-point  arith_type=Unsigned  n_bits=2  bin_pt=0  preci_type=Single  exp_width=8  frac_width=24  explicit_period=off  period=1  equ=P=C  opselect=C  inp2=PCIN>>17  opr=+  inp1=P  carry=CIN  dbl_ovrd=off  block_type=constant
  Constant1 : xbsIndex_r4/Constant  const=0  gui_display_data_type=Boolean  arith_type=Boolean  n_bits=16  bin_pt=14  preci_type=Single  exp_width=8  frac_width=24  explicit_period=on  period=8  equ=P=C  opselect=C  inp2=PCIN>>17  opr=+  inp1=P  carry=CIN  dbl_ovrd=off  block_type=constant
  Convert : xbsIndex_r4/Convert  gui_display_data_type=Fixed-point  arith_type="Signed  (2's comp)"  n_bits=16  bin_pt=14  float_type=Single  exp_bits=8  fraction_bits=24  quantization=Truncate  overflow=Saturate  en=off  latency=0  dbl_ovrd=off  pipeline=off  block_type=convert
  Convert1 : xbsIndex_r4/Convert  gui_display_data_type=Fixed-point  arith_type="Signed  (2's comp)"  n_bits=16  bin_pt=14  float_type=Single  exp_bits=8  fraction_bits=24  quantization=Truncate  overflow=Saturate  en=off  latency=0  dbl_ovrd=off  pipeline=off  block_type=convert
  Counter : xbsIndex_r4/Counter  cnt_type=Count Limited  cnt_to=19  operation=Up  start_count=0  cnt_by_val=1  arith_type=Unsigned  n_bits=5  bin_pt=0  load_pin=off  rst=on  en=on  explicit_period=off  period=1  dbl_ovrd=off  use_behavioral_HDL=off  implementation=Fabric  block_type=counter
  Delay : xbsIndex_r4/Delay  rst=off  infoeditControl=Selection of Reset will increase slice count due to use of real FFs and instead of SRLs  en=off  latency=1  dbl_ovrd=off  reg_retiming=off  block_type=delay
  Gateway Out{1..9} (9 alike) : xbsIndex_r4/Gateway Out  inherit_from_input=off  hdl_port=off  timing_constraint=None  locs_specified=off  LOCs={}  UseAsDAC=off  DACChannel='1'  block_type=gatewayout
  Inverter : xbsIndex_r4/Inverter  en=off  latency=0  dbl_ovrd=off  block_type=inv
  Logical1 : xbsIndex_r4/Logical  logical_function=AND  inputs=2  en=off  latency=0  precision=Full  arith_type=Unsigned  n_bits=8  bin_pt=2  align_bp=on  dbl_ovrd=off  block_type=logical
      settings: xl_area=[1 0 0 1 0 0 0]  block_version=VER_STRING_GOES_HERE
  Mult : xbsIndex_r4/Mult  precision=User Defined  arith_type="Signed  (2's comp)"  n_bits=16  bin_pt=15  quantization=Truncate  overflow=Wrap  en=off  latency=1  dbl_ovrd=off  use_behavioral_HDL=off  opt=Speed  use_embedded=on  optimum_pipeline=off  pipeline=on  use_rpm=on  placement_style=Triangular  block_type=mult
  Mult1 : xbsIndex_r4/Mult  precision=User Defined  arith_type="Signed  (2's comp)"  n_bits=16  bin_pt=15  quantization=Truncate  overflow=Wrap  en=off  latency=1  dbl_ovrd=off  use_behavioral_HDL=off  opt=Speed  use_embedded=on  optimum_pipeline=off  pipeline=on  use_rpm=on  placement_style=Triangular  block_type=mult
  ROM : xbsIndex_r4/ROM  depth=length(barker_seq20)  initVector=fliplr(barker_seq20)  distributed_mem=Block RAM  rst=off  init_reg=0  en=off  latency=1  gui_display_data_type=Fixed-point  arith_type="Signed  (2's comp)"  n_bits=16  bin_pt=15  preci_type=Single  exp_width=8  frac_width=24  dbl_ovrd=off  optimize=Area  use_rpm=on  block_type=sprom
  Real-Imag to Complex : RealImagToComplex
  Register : xbsIndex_r4/Register  init=0  rst=on  en=on  dbl_ovrd=off  block_type=register
  Register1 : xbsIndex_r4/Register  init=0  rst=on  en=on  dbl_ovrd=off  block_type=register
  Register2 : xbsIndex_r4/Register  init=0  rst=on  en=off  dbl_ovrd=off  block_type=register
  Register3 : xbsIndex_r4/Register  init=0  rst=on  en=off  dbl_ovrd=off  block_type=register
  Register{4..7} (4 alike) : xbsIndex_r4/Register  init=0  rst=off  en=off  dbl_ovrd=off  block_type=register
  Relational : xbsIndex_r4/Relational  mode=a=b  en=off  latency=0  dbl_ovrd=off  block_type=relational
  Scope : Scope  NumInputPorts=7
      settings: TimeRange=60000  YMin=-1~-1~-1~-1~-0.4~-0.5~0  YMax=1~1~0.5~1~0.4~1~1  SaveName=ScopeData4
  To Workspace : ToWorkspace  VariableName=simout  SampleTime=-1  SaveFormat=Array
  IQ Valid : Outport
  " I" : Outport  Port=2
  " Q" : Outport  Port=3
  tag scope: CS_DSSS_Despread_I=global, CS_DSSS_Despread_Q=global
  wires:
  I/1 -> Register4/1
  Counter/1 -> ROM/1, Relational/1
  ROM/1 -> Register6/1
  Mult/1 -> Accumulator/1
  Mult1/1 -> Accumulator1/1
  Constant/1 -> Relational/2
  Relational/1 -> Register7/1
  Inverter/1 -> Counter/1, Register3/2, Register2/2, Register/2, Register1/2
  Accumulator/1 -> Register/1, Gateway Out8/1
  Accumulator1/1 -> Register1/1, Gateway Out9/1
  Real-Imag to Complex/1 -> To Workspace/1
  Register2/1 -> Gateway Out2/1
  Register3/1 -> Gateway Out3/1
  Gateway Out2/1 -> Real-Imag to Complex/1
  Gateway Out3/1 -> Real-Imag to Complex/2
  Register/1 -> Convert/1, Gateway Out1/1
  Q/1 -> Register5/1
  Register1/1 -> Convert1/1, Gateway Out6/1
  Delay/1 -> Logical1/1
  Sym Start/1 -> Logical1/2, Inverter/1, Gateway Out7/1, Counter/2
  Convert/1 -> " I"/1, [CS_DSSS_Despread_I]
  Convert1/1 -> " Q"/1, [CS_DSSS_Despread_Q]
  Logical1/1 -> IQ Valid/1
  Gateway Out4/1 -> Scope/1
  Gateway Out5/1 -> Scope/2
  Gateway Out8/1 -> Scope/3
  Gateway Out9/1 -> Scope/4
  Gateway Out1/1 -> Scope/5
  Gateway Out6/1 -> Scope/6
  Register4/1 -> Mult/1, Register2/1, Gateway Out4/1
  Register5/1 -> Mult1/1
  Register6/1 -> Mult1/2, Mult/2, Register3/1, Gateway Out5/1
  Register7/1 -> Accumulator1/2, Register1/3, Register/3, Delay/1, Accumulator/2
  Gateway Out7/1 -> Scope/7

== system wlan_phy_rx_pmd/DSSS Rx/Demod/Descramble ==
  Bit : Inport
  Bit Valid : Inport  Port=2
  Convert : xbsIndex_r4/Convert  gui_display_data_type=Boolean  arith_type=Boolean  n_bits=16  bin_pt=14  float_type=Single  exp_bits=8  fraction_bits=24  quantization=Truncate  overflow=Wrap  en=off  latency=0  dbl_ovrd=off  pipeline=off  block_type=convert
  Convert1 : xbsIndex_r4/Convert  gui_display_data_type=Boolean  arith_type=Boolean  n_bits=16  bin_pt=14  float_type=Single  exp_bits=8  fraction_bits=24  quantization=Truncate  overflow=Wrap  en=off  latency=0  dbl_ovrd=off  pipeline=off  block_type=convert
  Convert2 : xbsIndex_r4/Convert  gui_display_data_type=Fixed-point  arith_type=Unsigned  n_bits=1  bin_pt=0  float_type=Single  exp_bits=8  fraction_bits=24  quantization=Truncate  overflow=Wrap  en=off  latency=0  dbl_ovrd=off  pipeline=off  block_type=convert
  Logical : xbsIndex_r4/Logical  logical_function=XOR  inputs=2  en=off  latency=0  precision=Full  arith_type=Unsigned  n_bits=8  bin_pt=2  align_bp=on  dbl_ovrd=off  block_type=logical
      settings: xl_area=[0, 0, 0, 0, 0, 0, 0]  block_version=VER_STRING_GOES_HERE
  Logical1 : xbsIndex_r4/Logical  logical_function=XOR  inputs=2  en=off  latency=0  precision=Full  arith_type=Unsigned  n_bits=8  bin_pt=2  align_bp=on  dbl_ovrd=off  block_type=logical
      settings: xl_area=[0, 0, 0, 0, 0, 0, 0]  block_version=VER_STRING_GOES_HERE
  Register{,1..6} (7 alike) : xbsIndex_r4/Register  init=1  rst=on  en=on  dbl_ovrd=off  block_type=register
      settings: xl_area=[0, 0, 0, 0, 0, 0, 0]  block_version=VER_STRING_GOES_HERE
  Register7 : xbsIndex_r4/Register  init=0  rst=off  en=off  dbl_ovrd=off  block_type=register
  Register8 : xbsIndex_r4/Register  init=0  rst=off  en=off  dbl_ovrd=off  block_type=register
  " Bit" : Outport
  " Bit Valid" : Outport  Port=2
  wires:
  [DSSS_RX_RESET] -> Convert/1
  Register6/1 -> Logical/1
  Register5/1 -> Register6/1
  Register4/1 -> Register5/1
  Register3/1 -> Register4/1, Logical/2
  Register2/1 -> Register3/1
  Register1/1 -> Register2/1
  Register/1 -> Register1/1
  Bit Valid/1 -> Convert1/1
  Convert/1 -> Register5/2, Register6/2, Register4/2, Register3/2, Register2/2, Register1/2, Register/2
  Convert1/1 -> Register5/3, Register6/3, Register8/1, Register4/3, Register3/3, Register2/3, Register1/3, Register/3
  Convert2/1 -> Register7/1
  Bit/1 -> Register/1, Logical1/1
  Logical/1 -> Logical1/2
  Logical1/1 -> Convert2/1
  Register7/1 -> " Bit"/1
  Register8/1 -> " Bit Valid"/1

== system wlan_phy_rx_pmd/DSSS Rx/Demod/Header CRC ==
  Bit : Inport
  Bit Valid : Inport  Port=2
  CRC LFSR : Subsystem
  Constant : xbsIndex_r4/Constant  const=32  gui_display_data_type=Fixed-point  arith_type=Unsigned  n_bits=6  bin_pt=0  preci_type=Single  exp_width=8  frac_width=24  explicit_period=off  period=1  equ=P=C  opselect=C  inp2=PCIN>>17  opr=+  inp1=P  carry=CIN  dbl_ovrd=off  block_type=constant
  Counter1 : xbsIndex_r4/Counter  cnt_type=Free Running  cnt_to=Inf  operation=Up  start_count=0  cnt_by_val=1  arith_type=Unsigned  n_bits=8  bin_pt=0  load_pin=off  rst=on  en=on  explicit_period=off  period=1  dbl_ovrd=off  use_behavioral_HDL=off  implementation=Fabric  block_type=counter
  Display : Display
  Gateway Out{1..6} (6 alike) : xbsIndex_r4/Gateway Out  inherit_from_input=off  hdl_port=off  timing_constraint=None  locs_specified=off  LOCs={}  UseAsDAC=off  DACChannel='1'  block_type=gatewayout
  Logical1 : xbsIndex_r4/Logical  logical_function=AND  inputs=2  en=off  latency=0  precision=Full  arith_type=Unsigned  n_bits=16  bin_pt=0  align_bp=on  dbl_ovrd=off  block_type=logical
  Register8 : xbsIndex_r4/Register  init=0  rst=off  en=on  dbl_ovrd=off  block_type=register
  Relational1 : xbsIndex_r4/Relational  mode=a<b  en=off  latency=0  dbl_ovrd=off  block_type=relational
  Scope : Scope  NumInputPorts=7
      settings: TimeRange=100000  YMin=-1~-1~-1~-0.5~0~0~0  YMax=1~1~1~1.5~150~1~1  SaveName=ScopeData3
  CRC Calc : Outport
  wires:
  [DSSS_RX_RESET] -> Counter1/1, Gateway Out6/1
  Counter1/1 -> Relational1/1, Gateway Out2/1
  Constant/1 -> Relational1/2
  Relational1/1 -> Logical1/2
  Bit/1 -> CRC LFSR/1(Bit), Register8/1
  CRC LFSR/1(CRC16) -> Gateway Out4/1, CRC Calc/1
  Gateway Out4/1 -> Display/1
  Gateway Out1/1 -> Scope/6
  Gateway Out5/1 -> Scope/7
  Gateway Out2/1 -> Scope/5
  Gateway Out3/1 -> Scope/4
  Register8/1 -> Gateway Out3/1
  Gateway Out6/1 -> Scope/1
  Bit Valid/1 -> Logical1/1, Gateway Out1/1
  Logical1/1 -> Counter1/2, CRC LFSR/2(Bit Valid), Gateway Out5/1, Register8/2

== system wlan_phy_rx_pmd/DSSS Rx/Demod/Sync ==
  note: Timeout in two stages:
      |
      | A) In the first MIN_ONES_TOT bits, at least MIN_ONES bits must be 1 (the DSSS preamble is 128 1's).
      |   Timeout and reset if this fails.
      |
      | -If (A) is satisfied, search for the SFD magic number in the first BITS_TO_SFD bits. Timeout and reset
      |   if no SFD is found.
  Bit : Inport
  Bit Valid : Inport  Port=2
  Convert1 : xbsIndex_r4/Convert  gui_display_data_type=Boolean  arith_type=Boolean  n_bits=1  bin_pt=0  float_type=Single  exp_bits=8  fraction_bits=24  quantization=Truncate  overflow=Wrap  en=off  latency=0  dbl_ovrd=off  pipeline=off  block_type=convert
  Convert2 : xbsIndex_r4/Convert  gui_display_data_type=Boolean  arith_type=Boolean  n_bits=1  bin_pt=0  float_type=Single  exp_bits=8  fraction_bits=24  quantization=Truncate  overflow=Wrap  en=off  latency=0  dbl_ovrd=off  pipeline=off  block_type=convert
  Counter1 : xbsIndex_r4/Counter  cnt_type=Free Running  cnt_to=Inf  operation=Up  start_count=0  cnt_by_val=1  arith_type=Unsigned  n_bits=8  bin_pt=0  load_pin=off  rst=on  en=on  explicit_period=off  period=1  dbl_ovrd=off  use_behavioral_HDL=off  implementation=Fabric  block_type=counter
  Counter2 : xbsIndex_r4/Counter  cnt_type=Free Running  cnt_to=Inf  operation=Up  start_count=0  cnt_by_val=1  arith_type=Unsigned  n_bits=8  bin_pt=0  load_pin=off  rst=on  en=on  explicit_period=off  period=1  dbl_ovrd=off  use_behavioral_HDL=off  implementation=Fabric  block_type=counter
  DSSS Sync : Scope  NumInputPorts=7
      settings: TimeRange=56900  YMin=0~0~0~0~0~-1~-1  YMax=1~1~1~70~45~1~1  SaveName=ScopeData2
  Display : Display
  Display1 : Display
  Display2 : Display
  Down Sample1 : xbsIndex_r4/Down Sample  sample_ratio=8  sample_phase="Last Value of Frame  (most efficient)"  en=off  latency=1  dbl_ovrd=off  block_type=dsamp
  Gateway Out{,1..8} (9 alike) : xbsIndex_r4/Gateway Out  inherit_from_input=off  hdl_port=off  timing_constraint=None  locs_specified=off  LOCs={}  UseAsDAC=off  DACChannel='1'  block_type=gatewayout
  Inverter : xbsIndex_r4/Inverter  en=off  latency=0  dbl_ovrd=off  block_type=inv
      settings: xl_area=[0 0 0 0 0 0 0]  block_version=VER_STRING_GOES_HERE
  Logical : xbsIndex_r4/Logical  logical_function=AND  inputs=2  en=off  latency=0  precision=Full  arith_type=Unsigned  n_bits=8  bin_pt=2  align_bp=on  dbl_ovrd=off  block_type=logical
      settings: xl_area=[0, 0, 0, 0, 0, 0, 0]  block_version=VER_STRING_GOES_HERE
  Logical1 : xbsIndex_r4/Logical  logical_function=AND  inputs=3  en=off  latency=0  precision=Full  arith_type=Unsigned  n_bits=8  bin_pt=2  align_bp=on  dbl_ovrd=off  block_type=logical
      settings: xl_area=[0, 0, 0, 0, 0, 0, 0]  block_version=VER_STRING_GOES_HERE
  Logical2 : xbsIndex_r4/Logical  logical_function=AND  inputs=2  en=off  latency=0  precision=Full  arith_type=Unsigned  n_bits=8  bin_pt=2  align_bp=on  dbl_ovrd=off  block_type=logical
      settings: xl_area=[0, 0, 0, 0, 0, 0, 0]  block_version=VER_STRING_GOES_HERE
  Logical3 : xbsIndex_r4/Logical  logical_function=OR  inputs=2  en=off  latency=0  precision=Full  arith_type=Unsigned  n_bits=8  bin_pt=2  align_bp=on  dbl_ovrd=off  block_type=logical
      settings: xl_area=[0, 0, 0, 0, 0, 0, 0]  block_version=VER_STRING_GOES_HERE
  Logical4 : xbsIndex_r4/Logical  logical_function=AND  inputs=2  en=off  latency=0  precision=Full  arith_type=Unsigned  n_bits=8  bin_pt=2  align_bp=on  dbl_ovrd=off  block_type=logical
      settings: xl_area=[0, 0, 0, 0, 0, 0, 0]  block_version=VER_STRING_GOES_HERE
  Register8 : xbsIndex_r4/Register  init=0  rst=off  en=on  dbl_ovrd=off  block_type=register
  Relational1 : xbsIndex_r4/Relational  mode=a>b  en=off  latency=0  dbl_ovrd=off  block_type=relational
  Relational2 : xbsIndex_r4/Relational  mode=a>b  en=off  latency=0  dbl_ovrd=off  block_type=relational
  Relational3 : xbsIndex_r4/Relational  mode=a>b  en=off  latency=0  dbl_ovrd=off  block_type=relational
  S-R Latch : Subsystem
  SFD Search : Subsystem
  Payload Bit : Outport
  " Bit Valid" : Outport  Port=2
  tag scope: DSSS_RX_TIMEOUT=global
  wires:
  Bit/1 -> SFD Search/1(Bit), Convert1/1, Payload Bit/1, Register8/1
  Bit Valid/1 -> SFD Search/2(Bit Valid), Logical/2, Counter1/2, Convert2/1, Gateway Out1/1, Register8/2
  SFD Search/1(Found) -> S-R Latch/1(S)
  [DSSS_RX_RESET] -> S-R Latch/2(R)
  S-R Latch/1(Q) -> Logical/1, Inverter/1, Gateway Out4/1
  Logical/1 -> " Bit Valid"/1
  Counter1/1 -> Relational1/1, Relational3/1, Gateway Out5/1
  Gateway Out/1 -> Display/1
  [DSSS_RX_RESET] -> Counter1/1, Counter2/1
  Gateway Out4/1 -> DSSS Sync/3  "SFD Found"
  Gateway Out5/1 -> DSSS Sync/4  "Bit Counter"
  Gateway Out1/1 -> DSSS Sync/2  "Bit Valid"
  Down Sample1/1 -> Relational1/2
  Gateway Out2/1 -> DSSS Sync/1  "Bit"
  Logical2/1 -> Counter2/2
  [regRx_DSSS_RX_TIMEOUT_BITS_TO_SFD] -> Down Sample1/1
  Counter2/1 -> Relational2/2, Gateway Out7/1
  [regRx_DSSS_RX_TIMEOUT_MIN_ONES] -> Relational2/1
  [regRx_DSSS_RX_TIMEOUT_MIN_ONES_TOT] -> Relational3/2
  Relational3/1 -> Logical1/2
  Relational2/1 -> Logical1/3
  Logical3/1 -> [DSSS_RX_TIMEOUT], Gateway Out8/1
  Inverter/1 -> Logical1/1, Logical4/1
  Logical1/1 -> Logical3/2
  [regRx_DSSS_RX_TIMEOUT_BITS_TO_SFD] -> Gateway Out/1
  Gateway Out3/1 -> Display1/1
  [regRx_DSSS_RX_TIMEOUT_MIN_ONES_TOT] -> Gateway Out3/1
  Gateway Out6/1 -> Display2/1
  [regRx_DSSS_RX_TIMEOUT_MIN_ONES] -> Gateway Out6/1
  Gateway Out7/1 -> DSSS Sync/5  "Ones Counter"
  Gateway Out8/1 -> DSSS Sync/6  "Timed Out"
  Convert2/1 -> Logical2/1
  Convert1/1 -> Logical2/2
  Register8/1 -> Gateway Out2/1
  Logical4/1 -> Logical3/1
  Relational1/1 -> Logical4/2

== system wlan_phy_rx_pmd/DSSS Rx/Reset Gen/Posedge ==
  D : Inport
  Delay : xbsIndex_r4/Delay  rst=off  infoeditControl=Selection of Reset will increase slice count due to use of real FFs and instead of SRLs  en=off  latency=1  dbl_ovrd=off  reg_retiming=off  block_type=delay
      settings: infoedit=Hardware notes: A delay line is a chain, each link of which is an SRL16 followed by a flip-flop. If register retiming is enabled, the delay line is a chain of flip-flops.  xl_area=[1 1 0 0 0 0 0]  block_version=VER_STRING_GOES_HERE
  Inverter : xbsIndex_r4/Inverter  en=off  latency=0  dbl_ovrd=off  block_type=inv
      settings: xl_area=[0 0 0 0 0 0 0]  block_version=VER_STRING_GOES_HERE
  Logical1 : xbsIndex_r4/Logical  logical_function=AND  inputs=2  en=off  latency=0  precision=Full  arith_type=Unsigned  n_bits=8  bin_pt=2  align_bp=on  dbl_ovrd=off  block_type=logical
      settings: xl_area=[1 0 0 1 0 0 0]  block_version=VER_STRING_GOES_HERE
  Q : Outport
  wires:
  D/1 -> Logical1/2, Delay/1
  Logical1/1 -> Q/1
  Inverter/1 -> Logical1/1
  Delay/1 -> Inverter/1
